simulink model "v01_aa_rii_16r4t11f_ver117sa"  (Simulink 7.6)
settings: Solver=ode45  StartTime=0.0  StopTime=128000  RelTol=1e-3
undefined symbols (not defined in the file or in its folder): sfp
libraries: xbsIndex_r4 x26961, monroe_library x154, xbsReplacements_r4 x78, simulink x32, dspsigops x32, casper_library_misc x30, xps_library x18
(part 18 of 176)

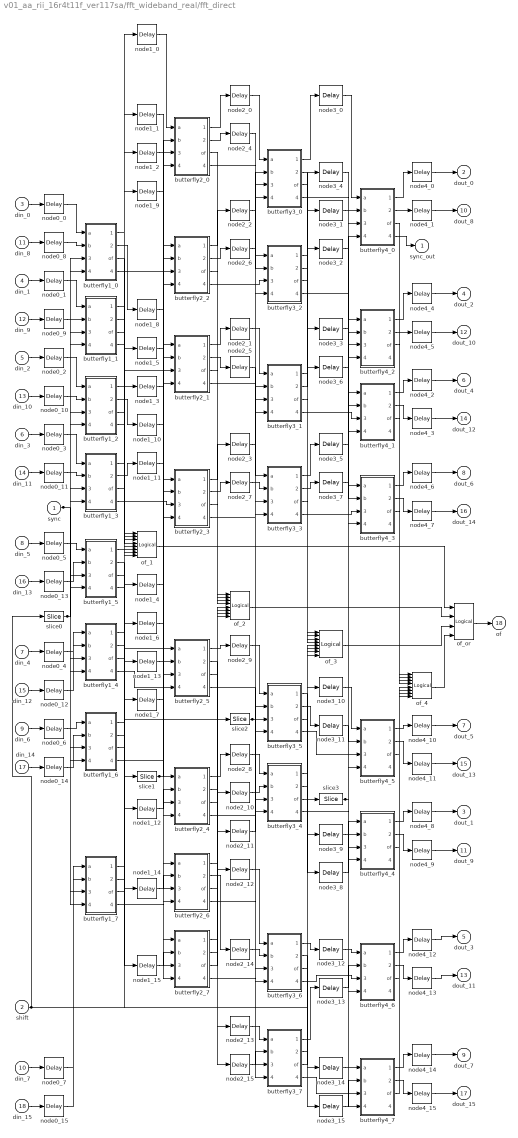
== system v01_aa_rii_16r4t11f_ver117sa/fft_wideband_real/fft_direct ==
  sync : Inport
  shift : Inport  Port=2
  din_0 : Inport  Port=3
  din_1 : Inport  Port=4
  din_2 : Inport  Port=5
  din_3 : Inport  Port=6
  din_4 : Inport  Port=7
  din_5 : Inport  Port=8
  din_6 : Inport  Port=9
  din_7 : Inport  Port=10
  din_8 : Inport  Port=11
  din_9 : Inport  Port=12
  din_10 : Inport  Port=13
  din_11 : Inport  Port=14
  din_12 : Inport  Port=15
  din_13 : Inport  Port=16
  din_14 : Inport  Port=17
  din_15 : Inport  Port=18
  butterfly1_0 : Subsystem
      settings: AttributesFormatString=|
      | twiddle_general_4mult
      | coeffs in BRAM
      | Bit growth:0
  butterfly1_1 : Subsystem
      settings: AttributesFormatString=|
      | twiddle_general_4mult
      | coeffs in BRAM
      | Bit growth:0
  butterfly1_2 : Subsystem
      settings: AttributesFormatString=|
      | twiddle_general_4mult
      | coeffs in BRAM
      | Bit growth:0
  butterfly1_3 : Subsystem
      settings: AttributesFormatString=|
      | twiddle_general_4mult
      | coeffs in BRAM
      | Bit growth:0
  butterfly1_4 : Subsystem
      settings: AttributesFormatString=|
      | twiddle_general_4mult
      | coeffs in BRAM
      | Bit growth:0
  butterfly1_5 : Subsystem
      settings: AttributesFormatString=|
      | twiddle_general_4mult
      | coeffs in BRAM
      | Bit growth:0
  butterfly1_6 : Subsystem
      settings: AttributesFormatString=|
      | twiddle_general_4mult
      | coeffs in BRAM
      | Bit growth:0
  butterfly1_7 : Subsystem
      settings: AttributesFormatString=|
      | twiddle_general_4mult
      | coeffs in BRAM
      | Bit growth:0
  butterfly2_0 : Subsystem
      settings: AttributesFormatString=|
      | twiddle_general_4mult
      | coeffs in BRAM
      | Bit growth:0
  butterfly2_1 : Subsystem
      settings: AttributesFormatString=|
      | twiddle_general_4mult
      | coeffs in BRAM
      | Bit growth:0
  butterfly2_2 : Subsystem
      settings: AttributesFormatString=|
      | twiddle_general_4mult
      | coeffs in BRAM
      | Bit growth:0
  butterfly2_3 : Subsystem
      settings: AttributesFormatString=|
      | twiddle_general_4mult
      | coeffs in BRAM
      | Bit growth:0
  butterfly2_4 : Subsystem
      settings: AttributesFormatString=|
      | twiddle_general_4mult
      | coeffs in BRAM
      | Bit growth:0
  butterfly2_5 : Subsystem
      settings: AttributesFormatString=|
      | twiddle_general_4mult
      | coeffs in BRAM
      | Bit growth:0
  butterfly2_6 : Subsystem
      settings: AttributesFormatString=|
      | twiddle_general_4mult
      | coeffs in BRAM
      | Bit growth:0
  butterfly2_7 : Subsystem
      settings: AttributesFormatString=|
      | twiddle_general_4mult
      | coeffs in BRAM
      | Bit growth:0
  butterfly3_0 : Subsystem
      settings: AttributesFormatString=|
      | twiddle_general_4mult
      | coeffs in BRAM
      | Bit growth:0
  butterfly3_1 : Subsystem
      settings: AttributesFormatString=|
      | twiddle_general_4mult
      | coeffs in BRAM
      | Bit growth:0
  butterfly3_2 : Subsystem
      settings: AttributesFormatString=|
      | twiddle_general_4mult
      | coeffs in BRAM
      | Bit growth:0
  butterfly3_3 : Subsystem
      settings: AttributesFormatString=|
      | twiddle_general_4mult
      | coeffs in BRAM
      | Bit growth:0
  butterfly3_4 : Subsystem
      settings: AttributesFormatString=|
      | twiddle_general_4mult
      | coeffs in BRAM
      | Bit growth:0
  butterfly3_5 : Subsystem
      settings: AttributesFormatString=|
      | twiddle_general_4mult
      | coeffs in BRAM
      | Bit growth:0
  butterfly3_6 : Subsystem
      settings: AttributesFormatString=|
      | twiddle_general_4mult
      | coeffs in BRAM
      | Bit growth:0
  butterfly3_7 : Subsystem
      settings: AttributesFormatString=|
      | twiddle_general_4mult
      | coeffs in BRAM
      | Bit growth:0
  butterfly4_0 : Subsystem
      settings: AttributesFormatString=|
      | twiddle_general_4mult
      | coeffs in BRAM
      | Bit growth:0
  butterfly4_1 : Subsystem
      settings: AttributesFormatString=|
      | twiddle_general_4mult
      | coeffs in BRAM
      | Bit growth:0
  butterfly4_2 : Subsystem
      settings: AttributesFormatString=|
      | twiddle_general_4mult
      | coeffs in BRAM
      | Bit growth:0
  butterfly4_3 : Subsystem
      settings: AttributesFormatString=|
      | twiddle_general_4mult
      | coeffs in BRAM
      | Bit growth:0
  butterfly4_4 : Subsystem
      settings: AttributesFormatString=|
      | twiddle_general_4mult
      | coeffs in BRAM
      | Bit growth:0
  butterfly4_5 : Subsystem
      settings: AttributesFormatString=|
      | twiddle_general_4mult
      | coeffs in BRAM
      | Bit growth:0
  butterfly4_6 : Subsystem
      settings: AttributesFormatString=|
      | twiddle_general_4mult
      | coeffs in BRAM
      | Bit growth:0
  butterfly4_7 : Subsystem
      settings: AttributesFormatString=|
      | twiddle_general_4mult
      | coeffs in BRAM
      | Bit growth:0
  node0_{0..15} (16 alike) : xbsIndex_r4/Delay  rst=off  infoeditControl=Selection of Reset will increase slice count due to use of real FFs and instead of SRLs  en=off  latency=0  dbl_ovrd=off  reg_retiming=off  block_type=delay
  node1_{0..15} (16 alike) : xbsIndex_r4/Delay  rst=off  infoeditControl=Selection of Reset will increase slice count due to use of real FFs and instead of SRLs  en=off  latency=0  dbl_ovrd=off  reg_retiming=off  block_type=delay
  node2_{0..15} (16 alike) : xbsIndex_r4/Delay  rst=off  infoeditControl=Selection of Reset will increase slice count due to use of real FFs and instead of SRLs  en=off  latency=0  dbl_ovrd=off  reg_retiming=off  block_type=delay
  node3_{0..15} (16 alike) : xbsIndex_r4/Delay  rst=off  infoeditControl=Selection of Reset will increase slice count due to use of real FFs and instead of SRLs  en=off  latency=0  dbl_ovrd=off  reg_retiming=off  block_type=delay
  node4_{0..15} (16 alike) : xbsIndex_r4/Delay  rst=off  infoeditControl=Selection of Reset will increase slice count due to use of real FFs and instead of SRLs  en=off  latency=0  dbl_ovrd=off  reg_retiming=off  block_type=delay
  of_{1..4} (4 alike) : xbsIndex_r4/Logical  logical_function=OR  inputs=8  en=off  latency=1  precision=Full  arith_type=Unsigned  n_bits=16  bin_pt=0  align_bp=on  dbl_ovrd=off  block_type=logical
  of_or : xbsIndex_r4/Logical  logical_function=OR  inputs=4  en=off  latency=0  precision=Full  arith_type=Unsigned  n_bits=16  bin_pt=0  align_bp=on  dbl_ovrd=off  block_type=logical
  slice0 : xbsIndex_r4/Slice  nbits=1  boolean_output=on  mode=Lower Bit Location + Width  bit1=0  base1=MSB of Input  bit0=0  base0=LSB of Input  dbl_ovrd=off  block_type=slice
  slice1 : xbsIndex_r4/Slice  nbits=1  boolean_output=on  mode=Lower Bit Location + Width  bit1=0  base1=MSB of Input  bit0=1  base0=LSB of Input  dbl_ovrd=off  block_type=slice
  slice2 : xbsIndex_r4/Slice  nbits=1  boolean_output=on  mode=Lower Bit Location + Width  bit1=0  base1=MSB of Input  bit0=2  base0=LSB of Input  dbl_ovrd=off  block_type=slice
  slice3 : xbsIndex_r4/Slice  nbits=1  boolean_output=on  mode=Lower Bit Location + Width  bit1=0  base1=MSB of Input  bit0=3  base0=LSB of Input  dbl_ovrd=off  block_type=slice
  sync_out : Outport
  dout_0 : Outport  Port=2
  dout_1 : Outport  Port=3
  dout_2 : Outport  Port=4
  dout_3 : Outport  Port=5
  dout_4 : Outport  Port=6
  dout_5 : Outport  Port=7
  dout_6 : Outport  Port=8
  dout_7 : Outport  Port=9
  dout_8 : Outport  Port=10
  dout_9 : Outport  Port=11
  dout_10 : Outport  Port=12
  dout_11 : Outport  Port=13
  dout_12 : Outport  Port=14
  dout_13 : Outport  Port=15
  dout_14 : Outport  Port=16
  dout_15 : Outport  Port=17
  of : Outport  Port=18
  wires:
  shift/1 -> slice3/1, slice2/1, slice1/1, slice0/1
  sync/1 -> butterfly1_7/3(sync), butterfly1_6/3(sync), butterfly1_5/3(sync), butterfly1_4/3(sync), butterfly1_3/3(sync), butterfly1_2/3(sync), butterfly1_1/3(sync), butterfly1_0/3(sync)
  slice0/1 -> butterfly1_7/4(shift), butterfly1_6/4(shift), butterfly1_5/4(shift), butterfly1_4/4(shift), butterfly1_3/4(shift), butterfly1_2/4(shift), butterfly1_1/4(shift), butterfly1_0/4(shift)
  slice1/1 -> butterfly2_7/4(shift), butterfly2_6/4(shift), butterfly2_5/4(shift), butterfly2_4/4(shift), butterfly2_3/4(shift), butterfly2_2/4(shift), butterfly2_1/4(shift), butterfly2_0/4(shift)
  slice2/1 -> butterfly3_7/4(shift), butterfly3_6/4(shift), butterfly3_5/4(shift), butterfly3_4/4(shift), butterfly3_3/4(shift), butterfly3_2/4(shift), butterfly3_1/4(shift), butterfly3_0/4(shift)
  slice3/1 -> butterfly4_7/4(shift), butterfly4_6/4(shift), butterfly4_5/4(shift), butterfly4_4/4(shift), butterfly4_3/4(shift), butterfly4_2/4(shift), butterfly4_1/4(shift), butterfly4_0/4(shift)
  butterfly4_0/4(sync_out) -> sync_out/1
  of_or/1 -> of/1
  of_4/1 -> of_or/4
  of_3/1 -> of_or/3
  of_2/1 -> of_or/2
  of_1/1 -> of_or/1
  butterfly4_7/3(of) -> of_4/8
  butterfly4_6/3(of) -> of_4/7
  butterfly4_5/3(of) -> of_4/6
  butterfly4_4/3(of) -> of_4/5
  butterfly4_3/3(of) -> of_4/4
  butterfly4_2/3(of) -> of_4/3
  butterfly4_1/3(of) -> of_4/2
  butterfly4_0/3(of) -> of_4/1
  butterfly4_7/2(a-bw) -> node4_15/1
  butterfly4_7/1(a+bw) -> node4_14/1
  butterfly3_7/4(sync_out) -> butterfly4_7/3(sync)
  node3_15/1 -> butterfly4_7/2(b)
  node3_14/1 -> butterfly4_7/1(a)
  butterfly4_6/2(a-bw) -> node4_13/1
  butterfly4_6/1(a+bw) -> node4_12/1
  butterfly3_6/4(sync_out) -> butterfly4_6/3(sync)
  node3_13/1 -> butterfly4_6/2(b)
  node3_12/1 -> butterfly4_6/1(a)
  butterfly4_5/2(a-bw) -> node4_11/1
  butterfly4_5/1(a+bw) -> node4_10/1
  butterfly3_5/4(sync_out) -> butterfly4_5/3(sync)
  node3_11/1 -> butterfly4_5/2(b)
  node3_10/1 -> butterfly4_5/1(a)
  butterfly4_4/2(a-bw) -> node4_9/1
  butterfly4_4/1(a+bw) -> node4_8/1
  butterfly3_4/4(sync_out) -> butterfly4_4/3(sync)
  node3_9/1 -> butterfly4_4/2(b)
  node3_8/1 -> butterfly4_4/1(a)
  butterfly4_3/2(a-bw) -> node4_7/1
  butterfly4_3/1(a+bw) -> node4_6/1
  butterfly3_3/4(sync_out) -> butterfly4_3/3(sync)
  node3_7/1 -> butterfly4_3/2(b)
  node3_6/1 -> butterfly4_3/1(a)
  butterfly4_2/2(a-bw) -> node4_5/1
  butterfly4_2/1(a+bw) -> node4_4/1
  butterfly3_2/4(sync_out) -> butterfly4_2/3(sync)
  node3_5/1 -> butterfly4_2/2(b)
  node3_4/1 -> butterfly4_2/1(a)
  butterfly4_1/2(a-bw) -> node4_3/1
  butterfly4_1/1(a+bw) -> node4_2/1
  butterfly3_1/4(sync_out) -> butterfly4_1/3(sync)
  node3_3/1 -> butterfly4_1/2(b)
  node3_2/1 -> butterfly4_1/1(a)
  butterfly4_0/2(a-bw) -> node4_1/1
  butterfly4_0/1(a+bw) -> node4_0/1
  butterfly3_0/4(sync_out) -> butterfly4_0/3(sync)
  node3_1/1 -> butterfly4_0/2(b)
  node3_0/1 -> butterfly4_0/1(a)
  butterfly3_7/3(of) -> of_3/8
  butterfly3_6/3(of) -> of_3/7
  butterfly3_5/3(of) -> of_3/6
  butterfly3_4/3(of) -> of_3/5
  butterfly3_3/3(of) -> of_3/4
  butterfly3_2/3(of) -> of_3/3
  butterfly3_1/3(of) -> of_3/2
  butterfly3_0/3(of) -> of_3/1
  butterfly3_7/2(a-bw) -> node3_15/1
  butterfly3_7/1(a+bw) -> node3_13/1
  butterfly2_7/4(sync_out) -> butterfly3_7/3(sync)
  node2_15/1 -> butterfly3_7/2(b)
  node2_13/1 -> butterfly3_7/1(a)
  butterfly3_6/2(a-bw) -> node3_14/1
  butterfly3_6/1(a+bw) -> node3_12/1
  butterfly2_6/4(sync_out) -> butterfly3_6/3(sync)
  node2_14/1 -> butterfly3_6/2(b)
  node2_12/1 -> butterfly3_6/1(a)
  butterfly3_5/2(a-bw) -> node3_11/1
  butterfly3_5/1(a+bw) -> node3_9/1
  butterfly2_5/4(sync_out) -> butterfly3_5/3(sync)
  node2_11/1 -> butterfly3_5/2(b)
  node2_9/1 -> butterfly3_5/1(a)
  butterfly3_4/2(a-bw) -> node3_10/1
  butterfly3_4/1(a+bw) -> node3_8/1
  butterfly2_4/4(sync_out) -> butterfly3_4/3(sync)
  node2_10/1 -> butterfly3_4/2(b)
  node2_8/1 -> butterfly3_4/1(a)
  butterfly3_3/2(a-bw) -> node3_7/1
  butterfly3_3/1(a+bw) -> node3_5/1
  butterfly2_3/4(sync_out) -> butterfly3_3/3(sync)
  node2_7/1 -> butterfly3_3/2(b)
  node2_5/1 -> butterfly3_3/1(a)
  butterfly3_2/2(a-bw) -> node3_6/1
  butterfly3_2/1(a+bw) -> node3_4/1
  butterfly2_2/4(sync_out) -> butterfly3_2/3(sync)
  node2_6/1 -> butterfly3_2/2(b)
  node2_4/1 -> butterfly3_2/1(a)
  butterfly3_1/2(a-bw) -> node3_3/1
  butterfly3_1/1(a+bw) -> node3_1/1
  butterfly2_1/4(sync_out) -> butterfly3_1/3(sync)
  node2_3/1 -> butterfly3_1/2(b)
  node2_1/1 -> butterfly3_1/1(a)
  butterfly3_0/2(a-bw) -> node3_2/1
  butterfly3_0/1(a+bw) -> node3_0/1
  butterfly2_0/4(sync_out) -> butterfly3_0/3(sync)
  node2_2/1 -> butterfly3_0/2(b)
  node2_0/1 -> butterfly3_0/1(a)
  butterfly2_7/3(of) -> of_2/8
  butterfly2_6/3(of) -> of_2/7
  butterfly2_5/3(of) -> of_2/6
  butterfly2_4/3(of) -> of_2/5
  butterfly2_3/3(of) -> of_2/4
  butterfly2_2/3(of) -> of_2/3
  butterfly2_1/3(of) -> of_2/2
  butterfly2_0/3(of) -> of_2/1
  butterfly2_7/2(a-bw) -> node2_15/1
  butterfly2_7/1(a+bw) -> node2_11/1
  butterfly1_7/4(sync_out) -> butterfly2_7/3(sync)
  node1_15/1 -> butterfly2_7/2(b)
  node1_11/1 -> butterfly2_7/1(a)
  butterfly2_6/2(a-bw) -> node2_14/1
  butterfly2_6/1(a+bw) -> node2_10/1
  butterfly1_6/4(sync_out) -> butterfly2_6/3(sync)
  node1_14/1 -> butterfly2_6/2(b)
  node1_10/1 -> butterfly2_6/1(a)
  butterfly2_5/2(a-bw) -> node2_13/1
  butterfly2_5/1(a+bw) -> node2_9/1
  butterfly1_5/4(sync_out) -> butterfly2_5/3(sync)
  node1_13/1 -> butterfly2_5/2(b)
  node1_9/1 -> butterfly2_5/1(a)
  butterfly2_4/2(a-bw) -> node2_12/1
  butterfly2_4/1(a+bw) -> node2_8/1
  butterfly1_4/4(sync_out) -> butterfly2_4/3(sync)
  node1_12/1 -> butterfly2_4/2(b)
  node1_8/1 -> butterfly2_4/1(a)
  butterfly2_3/2(a-bw) -> node2_7/1
  butterfly2_3/1(a+bw) -> node2_3/1
  butterfly1_3/4(sync_out) -> butterfly2_3/3(sync)
  node1_7/1 -> butterfly2_3/2(b)
  node1_3/1 -> butterfly2_3/1(a)
  butterfly2_2/2(a-bw) -> node2_6/1
  butterfly2_2/1(a+bw) -> node2_2/1
  butterfly1_2/4(sync_out) -> butterfly2_2/3(sync)
  node1_6/1 -> butterfly2_2/2(b)
  node1_2/1 -> butterfly2_2/1(a)
  butterfly2_1/2(a-bw) -> node2_5/1
  butterfly2_1/1(a+bw) -> node2_1/1
  butterfly1_1/4(sync_out) -> butterfly2_1/3(sync)
  node1_5/1 -> butterfly2_1/2(b)
  node1_1/1 -> butterfly2_1/1(a)
  butterfly2_0/2(a-bw) -> node2_4/1
  butterfly2_0/1(a+bw) -> node2_0/1
  butterfly1_0/4(sync_out) -> butterfly2_0/3(sync)
  node1_4/1 -> butterfly2_0/2(b)
  node1_0/1 -> butterfly2_0/1(a)
  butterfly1_7/3(of) -> of_1/8
  butterfly1_6/3(of) -> of_1/7
  butterfly1_5/3(of) -> of_1/6
  butterfly1_4/3(of) -> of_1/5
  butterfly1_3/3(of) -> of_1/4
  butterfly1_2/3(of) -> of_1/3
  butterfly1_1/3(of) -> of_1/2
  butterfly1_0/3(of) -> of_1/1
  butterfly1_7/2(a-bw) -> node1_15/1
  butterfly1_7/1(a+bw) -> node1_7/1
  node0_15/1 -> butterfly1_7/2(b)
  node0_7/1 -> butterfly1_7/1(a)
  butterfly1_6/2(a-bw) -> node1_14/1
  butterfly1_6/1(a+bw) -> node1_6/1
  node0_14/1 -> butterfly1_6/2(b)
  node0_6/1 -> butterfly1_6/1(a)
  butterfly1_5/2(a-bw) -> node1_13/1
  butterfly1_5/1(a+bw) -> node1_5/1
  node0_13/1 -> butterfly1_5/2(b)
  node0_5/1 -> butterfly1_5/1(a)
  butterfly1_4/2(a-bw) -> node1_12/1
  butterfly1_4/1(a+bw) -> node1_4/1
  node0_12/1 -> butterfly1_4/2(b)
  node0_4/1 -> butterfly1_4/1(a)
  butterfly1_3/2(a-bw) -> node1_11/1
  butterfly1_3/1(a+bw) -> node1_3/1
  node0_11/1 -> butterfly1_3/2(b)
  node0_3/1 -> butterfly1_3/1(a)
  butterfly1_2/2(a-bw) -> node1_10/1
  butterfly1_2/1(a+bw) -> node1_2/1
  node0_10/1 -> butterfly1_2/2(b)
  node0_2/1 -> butterfly1_2/1(a)
  butterfly1_1/2(a-bw) -> node1_9/1
  butterfly1_1/1(a+bw) -> node1_1/1
  node0_9/1 -> butterfly1_1/2(b)
  node0_1/1 -> butterfly1_1/1(a)
  butterfly1_0/2(a-bw) -> node1_8/1
  butterfly1_0/1(a+bw) -> node1_0/1
  node0_8/1 -> butterfly1_0/2(b)
  node0_0/1 -> butterfly1_0/1(a)
  node4_15/1 -> dout_15/1
  node4_14/1 -> dout_7/1
  node4_13/1 -> dout_11/1
  node4_12/1 -> dout_3/1
  node4_11/1 -> dout_13/1
  node4_10/1 -> dout_5/1
  node4_9/1 -> dout_9/1
  node4_8/1 -> dout_1/1
  node4_7/1 -> dout_14/1
  node4_6/1 -> dout_6/1
  node4_5/1 -> dout_10/1
  node4_4/1 -> dout_2/1
  node4_3/1 -> dout_12/1
  node4_2/1 -> dout_4/1
  node4_1/1 -> dout_8/1
  node4_0/1 -> dout_0/1
  din_15/1 -> node0_15/1
  din_14/1 -> node0_14/1
  din_13/1 -> node0_13/1
  din_12/1 -> node0_12/1
  din_11/1 -> node0_11/1
  din_10/1 -> node0_10/1
  din_9/1 -> node0_9/1
  din_8/1 -> node0_8/1
  din_7/1 -> node0_7/1
  din_6/1 -> node0_6/1
  din_5/1 -> node0_5/1
  din_4/1 -> node0_4/1
  din_3/1 -> node0_3/1
  din_2/1 -> node0_2/1
  din_1/1 -> node0_1/1
  din_0/1 -> node0_0/1

== system v01_aa_rii_16r4t11f_ver117sa/fft_wideband_real/fft_unscrambler ==
  sync : Inport
  In1 : Inport  Port=2
  In2 : Inport  Port=3
  In3 : Inport  Port=4
  In4 : Inport  Port=5
  In5 : Inport  Port=6
  In6 : Inport  Port=7
  In7 : Inport  Port=8
  In8 : Inport  Port=9
  const : xbsIndex_r4/Constant  const=1  gui_display_data_type=Fixed-point  arith_type=Boolean  n_bits=1  bin_pt=0  preci_type=Single  exp_width=8  frac_width=24  explicit_period=on  period=1  equ=P=C  opselect=C  inp2=PCIN>>17  opr=+  inp1=P  carry=CIN  dbl_ovrd=off  block_type=constant
  reorder1 : Subsystem
      settings: AttributesFormatString=order=7
  square_transposer1 : Subsystem
      settings: AttributesFormatString=n_inputs=3
  sync_out : Outport
  Out1 : Outport  Port=2
  Out2 : Outport  Port=3
  Out3 : Outport  Port=4
  Out4 : Outport  Port=5
  Out5 : Outport  Port=6
  Out6 : Outport  Port=7
  Out7 : Outport  Port=8
  Out8 : Outport  Port=9
  wires:
  square_transposer1/9(Out8) -> reorder1/10(din7)  "xlsub2_reorder_In8"
  square_transposer1/8(Out7) -> reorder1/9(din6)  "xlsub2_reorder_In7"
  square_transposer1/7(Out6) -> reorder1/8(din5)  "xlsub2_reorder_In6"
  square_transposer1/6(Out5) -> reorder1/7(din4)  "xlsub2_reorder_In5"
  square_transposer1/5(Out4) -> reorder1/6(din3)  "xlsub2_reorder_In4"
  square_transposer1/4(Out3) -> reorder1/5(din2)  "xlsub2_reorder_In3"
  square_transposer1/3(Out2) -> reorder1/4(din1)  "xlsub2_reorder_In2"
  square_transposer1/2(Out1) -> reorder1/3(din0)  "xlsub2_reorder_In1"
  square_transposer1/1(sync_out) -> reorder1/1(sync)
  In8/1 -> square_transposer1/9(In8)
  In7/1 -> square_transposer1/8(In7)
  In6/1 -> square_transposer1/7(In6)
  In5/1 -> square_transposer1/6(In5)
  In4/1 -> square_transposer1/5(In4)
  In3/1 -> square_transposer1/4(In3)
  In2/1 -> square_transposer1/3(In2)
  In1/1 -> square_transposer1/2(In1)
  sync/1 -> square_transposer1/1(sync)
  reorder1/10(dout7) -> Out8/1
  reorder1/9(dout6) -> Out7/1
  reorder1/8(dout5) -> Out6/1
  reorder1/7(dout4) -> Out5/1
  reorder1/6(dout3) -> Out4/1
  reorder1/5(dout2) -> Out3/1
  reorder1/4(dout1) -> Out2/1
  reorder1/3(dout0) -> Out1/1
  reorder1/1(sync_out) -> sync_out/1
  const/1 -> reorder1/2(en)

== system v01_aa_rii_16r4t11f_ver117sa/fft_wideband_real/in_del_4x0pol1 ==
  d : Inport
  q : Outport
  wires:
  d/1 -> q/1

== system v01_aa_rii_16r4t11f_ver117sa/fft_wideband_real/in_del_4x0pol2 ==
  d : Inport
  q : Outport
  wires:
  d/1 -> q/1

== system v01_aa_rii_16r4t11f_ver117sa/fft_wideband_real/in_del_4x0pol3 ==
  d : Inport
  q : Outport
  wires:
  d/1 -> q/1

== system v01_aa_rii_16r4t11f_ver117sa/fft_wideband_real/in_del_4x0pol4 ==
  d : Inport
  q : Outport
  wires:
  d/1 -> q/1

== system v01_aa_rii_16r4t11f_ver117sa/fft_wideband_real/in_del_4x1pol1 ==
  d : Inport
  q : Outport
  wires:
  d/1 -> q/1

== system v01_aa_rii_16r4t11f_ver117sa/fft_wideband_real/in_del_4x1pol2 ==
  d : Inport
  q : Outport
  wires:
  d/1 -> q/1

== system v01_aa_rii_16r4t11f_ver117sa/fft_wideband_real/in_del_4x1pol3 ==
  d : Inport
  q : Outport
  wires:
  d/1 -> q/1

== system v01_aa_rii_16r4t11f_ver117sa/fft_wideband_real/in_del_4x1pol4 ==
  d : Inport
  q : Outport
  wires:
  d/1 -> q/1

== system v01_aa_rii_16r4t11f_ver117sa/fft_wideband_real/in_del_4x2pol1 ==
  d : Inport
  q : Outport
  wires:
  d/1 -> q/1

== system v01_aa_rii_16r4t11f_ver117sa/fft_wideband_real/in_del_4x2pol2 ==
  d : Inport
  q : Outport
  wires:
  d/1 -> q/1

== system v01_aa_rii_16r4t11f_ver117sa/fft_wideband_real/in_del_4x2pol3 ==
  d : Inport
  q : Outport
  wires:
  d/1 -> q/1

== system v01_aa_rii_16r4t11f_ver117sa/fft_wideband_real/in_del_4x2pol4 ==
  d : Inport
  q : Outport
  wires:
  d/1 -> q/1

== system v01_aa_rii_16r4t11f_ver117sa/fft_wideband_real/in_del_4x3pol1 ==
  d : Inport
  q : Outport
  wires:
  d/1 -> q/1

== system v01_aa_rii_16r4t11f_ver117sa/fft_wideband_real/in_del_4x3pol2 ==
  d : Inport
  q : Outport
  wires:
  d/1 -> q/1

== system v01_aa_rii_16r4t11f_ver117sa/fft_wideband_real/in_del_4x3pol3 ==
  d : Inport
  q : Outport
  wires:
  d/1 -> q/1

== system v01_aa_rii_16r4t11f_ver117sa/fft_wideband_real/in_del_4x3pol4 ==
  d : Inport
  q : Outport
  wires:
  d/1 -> q/1

== system v01_aa_rii_16r4t11f_ver117sa/fft_wideband_real/in_del_sync_4x0 ==
  d : Inport
  q : Outport
  wires:
  d/1 -> q/1

== system v01_aa_rii_16r4t11f_ver117sa/fft_wideband_real/in_del_sync_4x1 ==
  d : Inport
  q : Outport
  wires:
  d/1 -> q/1

== system v01_aa_rii_16r4t11f_ver117sa/fft_wideband_real/in_del_sync_4x2 ==
  d : Inport
  q : Outport
  wires:
  d/1 -> q/1

== system v01_aa_rii_16r4t11f_ver117sa/fft_wideband_real/in_del_sync_4x3 ==
  d : Inport
  q : Outport
  wires:
  d/1 -> q/1

== system v01_aa_rii_16r4t11f_ver117sa/fft_wideband_real/out_del_4x0pol1 ==
  d : Inport
  q : Outport
  wires:
  d/1 -> q/1

== system v01_aa_rii_16r4t11f_ver117sa/fft_wideband_real/out_del_4x0pol2 ==
  d : Inport
  q : Outport
  wires:
  d/1 -> q/1

== system v01_aa_rii_16r4t11f_ver117sa/fft_wideband_real/out_del_4x0pol3 ==
  d : Inport
  q : Outport
  wires:
  d/1 -> q/1

== system v01_aa_rii_16r4t11f_ver117sa/fft_wideband_real/out_del_4x0pol4 ==
  d : Inport
  q : Outport
  wires:
  d/1 -> q/1

== system v01_aa_rii_16r4t11f_ver117sa/fft_wideband_real/out_del_4x1pol1 ==
  d : Inport
  q : Outport
  wires:
  d/1 -> q/1

== system v01_aa_rii_16r4t11f_ver117sa/fft_wideband_real/out_del_4x1pol2 ==
  d : Inport
  q : Outport
  wires:
  d/1 -> q/1

== system v01_aa_rii_16r4t11f_ver117sa/fft_wideband_real/out_del_4x1pol3 ==
  d : Inport
  q : Outport
  wires:
  d/1 -> q/1

== system v01_aa_rii_16r4t11f_ver117sa/fft_wideband_real/out_del_4x1pol4 ==
  d : Inport
  q : Outport
  wires:
  d/1 -> q/1

== system v01_aa_rii_16r4t11f_ver117sa/fft_wideband_real/out_del_4x2pol1 ==
  d : Inport
  q : Outport
  wires:
  d/1 -> q/1

== system v01_aa_rii_16r4t11f_ver117sa/fft_wideband_real/out_del_4x2pol2 ==
  d : Inport
  q : Outport
  wires:
  d/1 -> q/1

== system v01_aa_rii_16r4t11f_ver117sa/fft_wideband_real/out_del_4x2pol3 ==
  d : Inport
  q : Outport
  wires:
  d/1 -> q/1

== system v01_aa_rii_16r4t11f_ver117sa/fft_wideband_real/out_del_4x2pol4 ==
  d : Inport
  q : Outport
  wires:
  d/1 -> q/1

== system v01_aa_rii_16r4t11f_ver117sa/fft_wideband_real/out_del_4x3pol1 ==
  d : Inport
  q : Outport
  wires:
  d/1 -> q/1

== system v01_aa_rii_16r4t11f_ver117sa/fft_wideband_real/out_del_4x3pol2 ==
  d : Inport
  q : Outport
  wires:
  d/1 -> q/1

== system v01_aa_rii_16r4t11f_ver117sa/fft_wideband_real/out_del_4x3pol3 ==
  d : Inport
  q : Outport
  wires:
  d/1 -> q/1

== system v01_aa_rii_16r4t11f_ver117sa/fft_wideband_real/out_del_4x3pol4 ==
  d : Inport
  q : Outport
  wires:
  d/1 -> q/1

== system v01_aa_rii_16r4t11f_ver117sa/fft_wideband_real/out_del_sync_4x0 ==
  d : Inport
  q : Outport
  wires:
  d/1 -> q/1

== system v01_aa_rii_16r4t11f_ver117sa/fft_wideband_real1/fft_biplex_real_4x0 ==
  sync : Inport
  shift : Inport  Port=2
  pol1 : Inport  Port=3
  pol2 : Inport  Port=4
  pol3 : Inport  Port=5
  pol4 : Inport  Port=6
  bi_real_unscr_4x : Subsystem
  biplex_core : Subsystem
      settings: AttributesFormatString=|
      | 7 stages
      | reduce logic
      | Virtex5
  ri_to_c_pol1 : Subsystem
  ri_to_c_pol2 : Subsystem
  sync_out : Outport
  pol1_out : Outport  Port=2
  pol2_out : Outport  Port=3
  pol3_out : Outport  Port=4
  pol4_out : Outport  Port=5
  of : Outport  Port=6
  wires:
  bi_real_unscr_4x/5(pol4_out) -> pol4_out/1
  bi_real_unscr_4x/4(pol3_out) -> pol3_out/1
  bi_real_unscr_4x/3(pol2_out) -> pol2_out/1
  bi_real_unscr_4x/2(pol1_out) -> pol1_out/1
  bi_real_unscr_4x/1(sync_out) -> sync_out/1
  biplex_core/3(out2) -> bi_real_unscr_4x/3(odd)
  biplex_core/2(out1) -> bi_real_unscr_4x/2(even)
  biplex_core/1(sync_out) -> bi_real_unscr_4x/1(sync)
  biplex_core/4(of) -> of/1
  ri_to_c_pol2/1(c) -> biplex_core/4(pol2)
  ri_to_c_pol1/1(c) -> biplex_core/3(pol1)
  shift/1 -> biplex_core/2(shift)
  sync/1 -> biplex_core/1(sync)
  pol4/1 -> ri_to_c_pol2/2(im)
  pol3/1 -> ri_to_c_pol2/1(re)
  pol2/1 -> ri_to_c_pol1/2(im)
  pol1/1 -> ri_to_c_pol1/1(re)

== system v01_aa_rii_16r4t11f_ver117sa/fft_wideband_real1/fft_biplex_real_4x1 ==
  sync : Inport
  shift : Inport  Port=2
  pol1 : Inport  Port=3
  pol2 : Inport  Port=4
  pol3 : Inport  Port=5
  pol4 : Inport  Port=6
  bi_real_unscr_4x : Subsystem
  biplex_core : Subsystem
      settings: AttributesFormatString=|
      | 7 stages
      | reduce logic
      | Virtex5
  ri_to_c_pol1 : Subsystem
  ri_to_c_pol2 : Subsystem
  sync_out : Outport
  pol1_out : Outport  Port=2
  pol2_out : Outport  Port=3
  pol3_out : Outport  Port=4
  pol4_out : Outport  Port=5
  of : Outport  Port=6
  wires:
  bi_real_unscr_4x/5(pol4_out) -> pol4_out/1
  bi_real_unscr_4x/4(pol3_out) -> pol3_out/1
  bi_real_unscr_4x/3(pol2_out) -> pol2_out/1
  bi_real_unscr_4x/2(pol1_out) -> pol1_out/1
  bi_real_unscr_4x/1(sync_out) -> sync_out/1
  biplex_core/3(out2) -> bi_real_unscr_4x/3(odd)
  biplex_core/2(out1) -> bi_real_unscr_4x/2(even)
  biplex_core/1(sync_out) -> bi_real_unscr_4x/1(sync)
  biplex_core/4(of) -> of/1
  ri_to_c_pol2/1(c) -> biplex_core/4(pol2)
  ri_to_c_pol1/1(c) -> biplex_core/3(pol1)
  shift/1 -> biplex_core/2(shift)
  sync/1 -> biplex_core/1(sync)
  pol4/1 -> ri_to_c_pol2/2(im)
  pol3/1 -> ri_to_c_pol2/1(re)
  pol2/1 -> ri_to_c_pol1/2(im)
  pol1/1 -> ri_to_c_pol1/1(re)

== system v01_aa_rii_16r4t11f_ver117sa/fft_wideband_real1/fft_biplex_real_4x2 ==
  sync : Inport
  shift : Inport  Port=2
  pol1 : Inport  Port=3
  pol2 : Inport  Port=4
  pol3 : Inport  Port=5
  pol4 : Inport  Port=6
  bi_real_unscr_4x : Subsystem
  biplex_core : Subsystem
      settings: AttributesFormatString=|
      | 7 stages
      | reduce logic
      | Virtex5
  ri_to_c_pol1 : Subsystem
  ri_to_c_pol2 : Subsystem
  sync_out : Outport
  pol1_out : Outport  Port=2
  pol2_out : Outport  Port=3
  pol3_out : Outport  Port=4
  pol4_out : Outport  Port=5
  of : Outport  Port=6
  wires:
  bi_real_unscr_4x/5(pol4_out) -> pol4_out/1
  bi_real_unscr_4x/4(pol3_out) -> pol3_out/1
  bi_real_unscr_4x/3(pol2_out) -> pol2_out/1
  bi_real_unscr_4x/2(pol1_out) -> pol1_out/1
  bi_real_unscr_4x/1(sync_out) -> sync_out/1
  biplex_core/3(out2) -> bi_real_unscr_4x/3(odd)
  biplex_core/2(out1) -> bi_real_unscr_4x/2(even)
  biplex_core/1(sync_out) -> bi_real_unscr_4x/1(sync)
  biplex_core/4(of) -> of/1
  ri_to_c_pol2/1(c) -> biplex_core/4(pol2)
  ri_to_c_pol1/1(c) -> biplex_core/3(pol1)
  shift/1 -> biplex_core/2(shift)
  sync/1 -> biplex_core/1(sync)
  pol4/1 -> ri_to_c_pol2/2(im)
  pol3/1 -> ri_to_c_pol2/1(re)
  pol2/1 -> ri_to_c_pol1/2(im)
  pol1/1 -> ri_to_c_pol1/1(re)

== system v01_aa_rii_16r4t11f_ver117sa/fft_wideband_real1/fft_biplex_real_4x3 ==
  sync : Inport
  shift : Inport  Port=2
  pol1 : Inport  Port=3
  pol2 : Inport  Port=4
  pol3 : Inport  Port=5
  pol4 : Inport  Port=6
  bi_real_unscr_4x : Subsystem
  biplex_core : Subsystem
      settings: AttributesFormatString=|
      | 7 stages
      | reduce logic
      | Virtex5
  ri_to_c_pol1 : Subsystem
  ri_to_c_pol2 : Subsystem
  sync_out : Outport
  pol1_out : Outport  Port=2
  pol2_out : Outport  Port=3
  pol3_out : Outport  Port=4
  pol4_out : Outport  Port=5
  of : Outport  Port=6
  wires:
  bi_real_unscr_4x/5(pol4_out) -> pol4_out/1
  bi_real_unscr_4x/4(pol3_out) -> pol3_out/1
  bi_real_unscr_4x/3(pol2_out) -> pol2_out/1
  bi_real_unscr_4x/2(pol1_out) -> pol1_out/1
  bi_real_unscr_4x/1(sync_out) -> sync_out/1
  biplex_core/3(out2) -> bi_real_unscr_4x/3(odd)
  biplex_core/2(out1) -> bi_real_unscr_4x/2(even)
  biplex_core/1(sync_out) -> bi_real_unscr_4x/1(sync)
  biplex_core/4(of) -> of/1
  ri_to_c_pol2/1(c) -> biplex_core/4(pol2)
  ri_to_c_pol1/1(c) -> biplex_core/3(pol1)
  shift/1 -> biplex_core/2(shift)
  sync/1 -> biplex_core/1(sync)
  pol4/1 -> ri_to_c_pol2/2(im)
  pol3/1 -> ri_to_c_pol2/1(re)
  pol2/1 -> ri_to_c_pol1/2(im)
  pol1/1 -> ri_to_c_pol1/1(re)

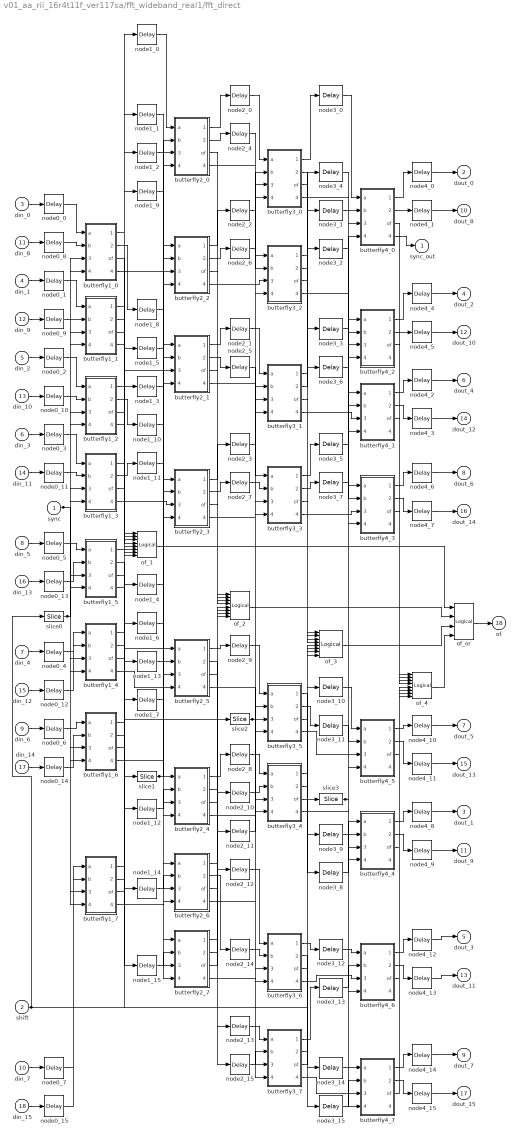
== system v01_aa_rii_16r4t11f_ver117sa/fft_wideband_real1/fft_direct ==
  sync : Inport
  shift : Inport  Port=2
  din_0 : Inport  Port=3
  din_1 : Inport  Port=4
  din_2 : Inport  Port=5
  din_3 : Inport  Port=6
  din_4 : Inport  Port=7
  din_5 : Inport  Port=8
  din_6 : Inport  Port=9
  din_7 : Inport  Port=10
  din_8 : Inport  Port=11
  din_9 : Inport  Port=12
  din_10 : Inport  Port=13
  din_11 : Inport  Port=14
  din_12 : Inport  Port=15
  din_13 : Inport  Port=16
  din_14 : Inport  Port=17
  din_15 : Inport  Port=18
  butterfly1_0 : Subsystem
      settings: AttributesFormatString=|
      | twiddle_general_4mult
      | coeffs in BRAM
      | Bit growth:0
  butterfly1_1 : Subsystem
      settings: AttributesFormatString=|
      | twiddle_general_4mult
      | coeffs in BRAM
      | Bit growth:0
  butterfly1_2 : Subsystem
      settings: AttributesFormatString=|
      | twiddle_general_4mult
      | coeffs in BRAM
      | Bit growth:0
  butterfly1_3 : Subsystem
      settings: AttributesFormatString=|
      | twiddle_general_4mult
      | coeffs in BRAM
      | Bit growth:0
  butterfly1_4 : Subsystem
      settings: AttributesFormatString=|
      | twiddle_general_4mult
      | coeffs in BRAM
      | Bit growth:0
  butterfly1_5 : Subsystem
      settings: AttributesFormatString=|
      | twiddle_general_4mult
      | coeffs in BRAM
      | Bit growth:0
  butterfly1_6 : Subsystem
      settings: AttributesFormatString=|
      | twiddle_general_4mult
      | coeffs in BRAM
      | Bit growth:0
  butterfly1_7 : Subsystem
      settings: AttributesFormatString=|
      | twiddle_general_4mult
      | coeffs in BRAM
      | Bit growth:0
  butterfly2_0 : Subsystem
      settings: AttributesFormatString=|
      | twiddle_general_4mult
      | coeffs in BRAM
      | Bit growth:0
  butterfly2_1 : Subsystem
      settings: AttributesFormatString=|
      | twiddle_general_4mult
      | coeffs in BRAM
      | Bit growth:0
  butterfly2_2 : Subsystem
      settings: AttributesFormatString=|
      | twiddle_general_4mult
      | coeffs in BRAM
      | Bit growth:0
  butterfly2_3 : Subsystem
      settings: AttributesFormatString=|
      | twiddle_general_4mult
      | coeffs in BRAM
      | Bit growth:0
  butterfly2_4 : Subsystem
      settings: AttributesFormatString=|
      | twiddle_general_4mult
      | coeffs in BRAM
      | Bit growth:0
  butterfly2_5 : Subsystem
      settings: AttributesFormatString=|
      | twiddle_general_4mult
      | coeffs in BRAM
      | Bit growth:0
  butterfly2_6 : Subsystem
      settings: AttributesFormatString=|
      | twiddle_general_4mult
      | coeffs in BRAM
      | Bit growth:0
  butterfly2_7 : Subsystem
      settings: AttributesFormatString=|
      | twiddle_general_4mult
      | coeffs in BRAM
      | Bit growth:0
  butterfly3_0 : Subsystem
      settings: AttributesFormatString=|
      | twiddle_general_4mult
      | coeffs in BRAM
      | Bit growth:0
  butterfly3_1 : Subsystem
      settings: AttributesFormatString=|
      | twiddle_general_4mult
      | coeffs in BRAM
      | Bit growth:0
  butterfly3_2 : Subsystem
      settings: AttributesFormatString=|
      | twiddle_general_4mult
      | coeffs in BRAM
      | Bit growth:0
  butterfly3_3 : Subsystem
      settings: AttributesFormatString=|
      | twiddle_general_4mult
      | coeffs in BRAM
      | Bit growth:0
  butterfly3_4 : Subsystem
      settings: AttributesFormatString=|
      | twiddle_general_4mult
      | coeffs in BRAM
      | Bit growth:0
  butterfly3_5 : Subsystem
      settings: AttributesFormatString=|
      | twiddle_general_4mult
      | coeffs in BRAM
      | Bit growth:0
  butterfly3_6 : Subsystem
      settings: AttributesFormatString=|
      | twiddle_general_4mult
      | coeffs in BRAM
      | Bit growth:0
  butterfly3_7 : Subsystem
      settings: AttributesFormatString=|
      | twiddle_general_4mult
      | coeffs in BRAM
      | Bit growth:0
  butterfly4_0 : Subsystem
      settings: AttributesFormatString=|
      | twiddle_general_4mult
      | coeffs in BRAM
      | Bit growth:0
  butterfly4_1 : Subsystem
      settings: AttributesFormatString=|
      | twiddle_general_4mult
      | coeffs in BRAM
      | Bit growth:0
  butterfly4_2 : Subsystem
      settings: AttributesFormatString=|
      | twiddle_general_4mult
      | coeffs in BRAM
      | Bit growth:0
  butterfly4_3 : Subsystem
      settings: AttributesFormatString=|
      | twiddle_general_4mult
      | coeffs in BRAM
      | Bit growth:0
  butterfly4_4 : Subsystem
      settings: AttributesFormatString=|
      | twiddle_general_4mult
      | coeffs in BRAM
      | Bit growth:0
  butterfly4_5 : Subsystem
      settings: AttributesFormatString=|
      | twiddle_general_4mult
      | coeffs in BRAM
      | Bit growth:0
  butterfly4_6 : Subsystem
      settings: AttributesFormatString=|
      | twiddle_general_4mult
      | coeffs in BRAM
      | Bit growth:0
  butterfly4_7 : Subsystem
      settings: AttributesFormatString=|
      | twiddle_general_4mult
      | coeffs in BRAM
      | Bit growth:0
  node0_{0..15} (16 alike) : xbsIndex_r4/Delay  rst=off  infoeditControl=Selection of Reset will increase slice count due to use of real FFs and instead of SRLs  en=off  latency=0  dbl_ovrd=off  reg_retiming=off  block_type=delay
  node1_{0..15} (16 alike) : xbsIndex_r4/Delay  rst=off  infoeditControl=Selection of Reset will increase slice count due to use of real FFs and instead of SRLs  en=off  latency=0  dbl_ovrd=off  reg_retiming=off  block_type=delay
  node2_{0..15} (16 alike) : xbsIndex_r4/Delay  rst=off  infoeditControl=Selection of Reset will increase slice count due to use of real FFs and instead of SRLs  en=off  latency=0  dbl_ovrd=off  reg_retiming=off  block_type=delay
  node3_{0..15} (16 alike) : xbsIndex_r4/Delay  rst=off  infoeditControl=Selection of Reset will increase slice count due to use of real FFs and instead of SRLs  en=off  latency=0  dbl_ovrd=off  reg_retiming=off  block_type=delay
  node4_{0..15} (16 alike) : xbsIndex_r4/Delay  rst=off  infoeditControl=Selection of Reset will increase slice count due to use of real FFs and instead of SRLs  en=off  latency=0  dbl_ovrd=off  reg_retiming=off  block_type=delay
  of_{1..4} (4 alike) : xbsIndex_r4/Logical  logical_function=OR  inputs=8  en=off  latency=1  precision=Full  arith_type=Unsigned  n_bits=16  bin_pt=0  align_bp=on  dbl_ovrd=off  block_type=logical
  of_or : xbsIndex_r4/Logical  logical_function=OR  inputs=4  en=off  latency=0  precision=Full  arith_type=Unsigned  n_bits=16  bin_pt=0  align_bp=on  dbl_ovrd=off  block_type=logical
  slice0 : xbsIndex_r4/Slice  nbits=1  boolean_output=on  mode=Lower Bit Location + Width  bit1=0  base1=MSB of Input  bit0=0  base0=LSB of Input  dbl_ovrd=off  block_type=slice
  slice1 : xbsIndex_r4/Slice  nbits=1  boolean_output=on  mode=Lower Bit Location + Width  bit1=0  base1=MSB of Input  bit0=1  base0=LSB of Input  dbl_ovrd=off  block_type=slice
  slice2 : xbsIndex_r4/Slice  nbits=1  boolean_output=on  mode=Lower Bit Location + Width  bit1=0  base1=MSB of Input  bit0=2  base0=LSB of Input  dbl_ovrd=off  block_type=slice
  slice3 : xbsIndex_r4/Slice  nbits=1  boolean_output=on  mode=Lower Bit Location + Width  bit1=0  base1=MSB of Input  bit0=3  base0=LSB of Input  dbl_ovrd=off  block_type=slice
  sync_out : Outport
  dout_0 : Outport  Port=2
  dout_1 : Outport  Port=3
  dout_2 : Outport  Port=4
  dout_3 : Outport  Port=5
  dout_4 : Outport  Port=6
  dout_5 : Outport  Port=7
  dout_6 : Outport  Port=8
  dout_7 : Outport  Port=9
  dout_8 : Outport  Port=10
  dout_9 : Outport  Port=11
  dout_10 : Outport  Port=12
  dout_11 : Outport  Port=13
  dout_12 : Outport  Port=14
  dout_13 : Outport  Port=15
  dout_14 : Outport  Port=16
  dout_15 : Outport  Port=17
  of : Outport  Port=18
  wires:
  shift/1 -> slice3/1, slice2/1, slice1/1, slice0/1
  sync/1 -> butterfly1_7/3(sync), butterfly1_6/3(sync), butterfly1_5/3(sync), butterfly1_4/3(sync), butterfly1_3/3(sync), butterfly1_2/3(sync), butterfly1_1/3(sync), butterfly1_0/3(sync)
  slice0/1 -> butterfly1_7/4(shift), butterfly1_6/4(shift), butterfly1_5/4(shift), butterfly1_4/4(shift), butterfly1_3/4(shift), butterfly1_2/4(shift), butterfly1_1/4(shift), butterfly1_0/4(shift)
  slice1/1 -> butterfly2_7/4(shift), butterfly2_6/4(shift), butterfly2_5/4(shift), butterfly2_4/4(shift), butterfly2_3/4(shift), butterfly2_2/4(shift), butterfly2_1/4(shift), butterfly2_0/4(shift)
  slice2/1 -> butterfly3_7/4(shift), butterfly3_6/4(shift), butterfly3_5/4(shift), butterfly3_4/4(shift), butterfly3_3/4(shift), butterfly3_2/4(shift), butterfly3_1/4(shift), butterfly3_0/4(shift)
  slice3/1 -> butterfly4_7/4(shift), butterfly4_6/4(shift), butterfly4_5/4(shift), butterfly4_4/4(shift), butterfly4_3/4(shift), butterfly4_2/4(shift), butterfly4_1/4(shift), butterfly4_0/4(shift)
  butterfly4_0/4(sync_out) -> sync_out/1
  of_or/1 -> of/1
  of_4/1 -> of_or/4
  of_3/1 -> of_or/3
  of_2/1 -> of_or/2
  of_1/1 -> of_or/1
  butterfly4_7/3(of) -> of_4/8
  butterfly4_6/3(of) -> of_4/7
  butterfly4_5/3(of) -> of_4/6
  butterfly4_4/3(of) -> of_4/5
  butterfly4_3/3(of) -> of_4/4
  butterfly4_2/3(of) -> of_4/3
  butterfly4_1/3(of) -> of_4/2
  butterfly4_0/3(of) -> of_4/1
  butterfly4_7/2(a-bw) -> node4_15/1
  butterfly4_7/1(a+bw) -> node4_14/1
  butterfly3_7/4(sync_out) -> butterfly4_7/3(sync)
  node3_15/1 -> butterfly4_7/2(b)
  node3_14/1 -> butterfly4_7/1(a)
  butterfly4_6/2(a-bw) -> node4_13/1
  butterfly4_6/1(a+bw) -> node4_12/1
  butterfly3_6/4(sync_out) -> butterfly4_6/3(sync)
  node3_13/1 -> butterfly4_6/2(b)
  node3_12/1 -> butterfly4_6/1(a)
  butterfly4_5/2(a-bw) -> node4_11/1
  butterfly4_5/1(a+bw) -> node4_10/1
  butterfly3_5/4(sync_out) -> butterfly4_5/3(sync)
  node3_11/1 -> butterfly4_5/2(b)
  node3_10/1 -> butterfly4_5/1(a)
  butterfly4_4/2(a-bw) -> node4_9/1
  butterfly4_4/1(a+bw) -> node4_8/1
  butterfly3_4/4(sync_out) -> butterfly4_4/3(sync)
  node3_9/1 -> butterfly4_4/2(b)
  node3_8/1 -> butterfly4_4/1(a)
  butterfly4_3/2(a-bw) -> node4_7/1
  butterfly4_3/1(a+bw) -> node4_6/1
  butterfly3_3/4(sync_out) -> butterfly4_3/3(sync)
  node3_7/1 -> butterfly4_3/2(b)
  node3_6/1 -> butterfly4_3/1(a)
  butterfly4_2/2(a-bw) -> node4_5/1
  butterfly4_2/1(a+bw) -> node4_4/1
  butterfly3_2/4(sync_out) -> butterfly4_2/3(sync)
  node3_5/1 -> butterfly4_2/2(b)
  node3_4/1 -> butterfly4_2/1(a)
  butterfly4_1/2(a-bw) -> node4_3/1
  butterfly4_1/1(a+bw) -> node4_2/1
  butterfly3_1/4(sync_out) -> butterfly4_1/3(sync)
  node3_3/1 -> butterfly4_1/2(b)
  node3_2/1 -> butterfly4_1/1(a)
  butterfly4_0/2(a-bw) -> node4_1/1
  butterfly4_0/1(a+bw) -> node4_0/1
  butterfly3_0/4(sync_out) -> butterfly4_0/3(sync)
  node3_1/1 -> butterfly4_0/2(b)
  node3_0/1 -> butterfly4_0/1(a)
  butterfly3_7/3(of) -> of_3/8
  butterfly3_6/3(of) -> of_3/7
  butterfly3_5/3(of) -> of_3/6
  butterfly3_4/3(of) -> of_3/5
  butterfly3_3/3(of) -> of_3/4
  butterfly3_2/3(of) -> of_3/3
  butterfly3_1/3(of) -> of_3/2
  butterfly3_0/3(of) -> of_3/1
  butterfly3_7/2(a-bw) -> node3_15/1
  butterfly3_7/1(a+bw) -> node3_13/1
  butterfly2_7/4(sync_out) -> butterfly3_7/3(sync)
  node2_15/1 -> butterfly3_7/2(b)
  node2_13/1 -> butterfly3_7/1(a)
  butterfly3_6/2(a-bw) -> node3_14/1
  butterfly3_6/1(a+bw) -> node3_12/1
  butterfly2_6/4(sync_out) -> butterfly3_6/3(sync)
  node2_14/1 -> butterfly3_6/2(b)
  node2_12/1 -> butterfly3_6/1(a)
  butterfly3_5/2(a-bw) -> node3_11/1
  butterfly3_5/1(a+bw) -> node3_9/1
  butterfly2_5/4(sync_out) -> butterfly3_5/3(sync)
  node2_11/1 -> butterfly3_5/2(b)
  node2_9/1 -> butterfly3_5/1(a)
  butterfly3_4/2(a-bw) -> node3_10/1
  butterfly3_4/1(a+bw) -> node3_8/1
  butterfly2_4/4(sync_out) -> butterfly3_4/3(sync)
  node2_10/1 -> butterfly3_4/2(b)
  node2_8/1 -> butterfly3_4/1(a)
  butterfly3_3/2(a-bw) -> node3_7/1
  butterfly3_3/1(a+bw) -> node3_5/1
  butterfly2_3/4(sync_out) -> butterfly3_3/3(sync)
  node2_7/1 -> butterfly3_3/2(b)
  node2_5/1 -> butterfly3_3/1(a)
  butterfly3_2/2(a-bw) -> node3_6/1
  butterfly3_2/1(a+bw) -> node3_4/1
  butterfly2_2/4(sync_out) -> butterfly3_2/3(sync)
  node2_6/1 -> butterfly3_2/2(b)
  node2_4/1 -> butterfly3_2/1(a)
  butterfly3_1/2(a-bw) -> node3_3/1
  butterfly3_1/1(a+bw) -> node3_1/1
  butterfly2_1/4(sync_out) -> butterfly3_1/3(sync)
  node2_3/1 -> butterfly3_1/2(b)
  node2_1/1 -> butterfly3_1/1(a)
  butterfly3_0/2(a-bw) -> node3_2/1
  butterfly3_0/1(a+bw) -> node3_0/1
  butterfly2_0/4(sync_out) -> butterfly3_0/3(sync)
  node2_2/1 -> butterfly3_0/2(b)
  node2_0/1 -> butterfly3_0/1(a)
  butterfly2_7/3(of) -> of_2/8
  butterfly2_6/3(of) -> of_2/7
  butterfly2_5/3(of) -> of_2/6
  butterfly2_4/3(of) -> of_2/5
  butterfly2_3/3(of) -> of_2/4
  butterfly2_2/3(of) -> of_2/3
  butterfly2_1/3(of) -> of_2/2
  butterfly2_0/3(of) -> of_2/1
  butterfly2_7/2(a-bw) -> node2_15/1
  butterfly2_7/1(a+bw) -> node2_11/1
  butterfly1_7/4(sync_out) -> butterfly2_7/3(sync)
  node1_15/1 -> butterfly2_7/2(b)
  node1_11/1 -> butterfly2_7/1(a)
  butterfly2_6/2(a-bw) -> node2_14/1
  butterfly2_6/1(a+bw) -> node2_10/1
  butterfly1_6/4(sync_out) -> butterfly2_6/3(sync)
  node1_14/1 -> butterfly2_6/2(b)
  node1_10/1 -> butterfly2_6/1(a)
  butterfly2_5/2(a-bw) -> node2_13/1
  butterfly2_5/1(a+bw) -> node2_9/1
  butterfly1_5/4(sync_out) -> butterfly2_5/3(sync)
  node1_13/1 -> butterfly2_5/2(b)
  node1_9/1 -> butterfly2_5/1(a)
  butterfly2_4/2(a-bw) -> node2_12/1
  butterfly2_4/1(a+bw) -> node2_8/1
  butterfly1_4/4(sync_out) -> butterfly2_4/3(sync)
  node1_12/1 -> butterfly2_4/2(b)
  node1_8/1 -> butterfly2_4/1(a)
  butterfly2_3/2(a-bw) -> node2_7/1
  butterfly2_3/1(a+bw) -> node2_3/1
  butterfly1_3/4(sync_out) -> butterfly2_3/3(sync)
  node1_7/1 -> butterfly2_3/2(b)
  node1_3/1 -> butterfly2_3/1(a)
  butterfly2_2/2(a-bw) -> node2_6/1
  butterfly2_2/1(a+bw) -> node2_2/1
  butterfly1_2/4(sync_out) -> butterfly2_2/3(sync)
  node1_6/1 -> butterfly2_2/2(b)
  node1_2/1 -> butterfly2_2/1(a)
  butterfly2_1/2(a-bw) -> node2_5/1
  butterfly2_1/1(a+bw) -> node2_1/1
  butterfly1_1/4(sync_out) -> butterfly2_1/3(sync)
  node1_5/1 -> butterfly2_1/2(b)
  node1_1/1 -> butterfly2_1/1(a)
  butterfly2_0/2(a-bw) -> node2_4/1
  butterfly2_0/1(a+bw) -> node2_0/1
  butterfly1_0/4(sync_out) -> butterfly2_0/3(sync)
  node1_4/1 -> butterfly2_0/2(b)
  node1_0/1 -> butterfly2_0/1(a)
  butterfly1_7/3(of) -> of_1/8
  butterfly1_6/3(of) -> of_1/7
  butterfly1_5/3(of) -> of_1/6
  butterfly1_4/3(of) -> of_1/5
  butterfly1_3/3(of) -> of_1/4
  butterfly1_2/3(of) -> of_1/3
  butterfly1_1/3(of) -> of_1/2
  butterfly1_0/3(of) -> of_1/1
  butterfly1_7/2(a-bw) -> node1_15/1
  butterfly1_7/1(a+bw) -> node1_7/1
  node0_15/1 -> butterfly1_7/2(b)
  node0_7/1 -> butterfly1_7/1(a)
  butterfly1_6/2(a-bw) -> node1_14/1
  butterfly1_6/1(a+bw) -> node1_6/1
  node0_14/1 -> butterfly1_6/2(b)
  node0_6/1 -> butterfly1_6/1(a)
  butterfly1_5/2(a-bw) -> node1_13/1
  butterfly1_5/1(a+bw) -> node1_5/1
  node0_13/1 -> butterfly1_5/2(b)
  node0_5/1 -> butterfly1_5/1(a)
  butterfly1_4/2(a-bw) -> node1_12/1
  butterfly1_4/1(a+bw) -> node1_4/1
  node0_12/1 -> butterfly1_4/2(b)
  node0_4/1 -> butterfly1_4/1(a)
  butterfly1_3/2(a-bw) -> node1_11/1
  butterfly1_3/1(a+bw) -> node1_3/1
  node0_11/1 -> butterfly1_3/2(b)
  node0_3/1 -> butterfly1_3/1(a)
  butterfly1_2/2(a-bw) -> node1_10/1
  butterfly1_2/1(a+bw) -> node1_2/1
  node0_10/1 -> butterfly1_2/2(b)
  node0_2/1 -> butterfly1_2/1(a)
  butterfly1_1/2(a-bw) -> node1_9/1
  butterfly1_1/1(a+bw) -> node1_1/1
  node0_9/1 -> butterfly1_1/2(b)
  node0_1/1 -> butterfly1_1/1(a)
  butterfly1_0/2(a-bw) -> node1_8/1
  butterfly1_0/1(a+bw) -> node1_0/1
  node0_8/1 -> butterfly1_0/2(b)
  node0_0/1 -> butterfly1_0/1(a)
  node4_15/1 -> dout_15/1
  node4_14/1 -> dout_7/1
  node4_13/1 -> dout_11/1
  node4_12/1 -> dout_3/1
  node4_11/1 -> dout_13/1
  node4_10/1 -> dout_5/1
  node4_9/1 -> dout_9/1
  node4_8/1 -> dout_1/1
  node4_7/1 -> dout_14/1
  node4_6/1 -> dout_6/1
  node4_5/1 -> dout_10/1
  node4_4/1 -> dout_2/1
  node4_3/1 -> dout_12/1
  node4_2/1 -> dout_4/1
  node4_1/1 -> dout_8/1
  node4_0/1 -> dout_0/1
  din_15/1 -> node0_15/1
  din_14/1 -> node0_14/1
  din_13/1 -> node0_13/1
  din_12/1 -> node0_12/1
  din_11/1 -> node0_11/1
  din_10/1 -> node0_10/1
  din_9/1 -> node0_9/1
  din_8/1 -> node0_8/1
  din_7/1 -> node0_7/1
  din_6/1 -> node0_6/1
  din_5/1 -> node0_5/1
  din_4/1 -> node0_4/1
  din_3/1 -> node0_3/1
  din_2/1 -> node0_2/1
  din_1/1 -> node0_1/1
  din_0/1 -> node0_0/1

== system v01_aa_rii_16r4t11f_ver117sa/fft_wideband_real1/fft_unscrambler ==
  sync : Inport
  In1 : Inport  Port=2
  In2 : Inport  Port=3
  In3 : Inport  Port=4
  In4 : Inport  Port=5
  In5 : Inport  Port=6
  In6 : Inport  Port=7
  In7 : Inport  Port=8
  In8 : Inport  Port=9
  const : xbsIndex_r4/Constant  const=1  gui_display_data_type=Fixed-point  arith_type=Boolean  n_bits=1  bin_pt=0  preci_type=Single  exp_width=8  frac_width=24  explicit_period=on  period=1  equ=P=C  opselect=C  inp2=PCIN>>17  opr=+  inp1=P  carry=CIN  dbl_ovrd=off  block_type=constant
  reorder1 : Subsystem
      settings: AttributesFormatString=order=7
  square_transposer1 : Subsystem
      settings: AttributesFormatString=n_inputs=3
  sync_out : Outport
  Out1 : Outport  Port=2
  Out2 : Outport  Port=3
  Out3 : Outport  Port=4
  Out4 : Outport  Port=5
  Out5 : Outport  Port=6
  Out6 : Outport  Port=7
  Out7 : Outport  Port=8
  Out8 : Outport  Port=9
  wires:
  square_transposer1/9(Out8) -> reorder1/10(din7)  "xlsub2_reorder_In8"
  square_transposer1/8(Out7) -> reorder1/9(din6)  "xlsub2_reorder_In7"
  square_transposer1/7(Out6) -> reorder1/8(din5)  "xlsub2_reorder_In6"
  square_transposer1/6(Out5) -> reorder1/7(din4)  "xlsub2_reorder_In5"
  square_transposer1/5(Out4) -> reorder1/6(din3)  "xlsub2_reorder_In4"
  square_transposer1/4(Out3) -> reorder1/5(din2)  "xlsub2_reorder_In3"
  square_transposer1/3(Out2) -> reorder1/4(din1)  "xlsub2_reorder_In2"
  square_transposer1/2(Out1) -> reorder1/3(din0)  "xlsub2_reorder_In1"
  square_transposer1/1(sync_out) -> reorder1/1(sync)
  In8/1 -> square_transposer1/9(In8)
  In7/1 -> square_transposer1/8(In7)
  In6/1 -> square_transposer1/7(In6)
  In5/1 -> square_transposer1/6(In5)
  In4/1 -> square_transposer1/5(In4)
  In3/1 -> square_transposer1/4(In3)
  In2/1 -> square_transposer1/3(In2)
  In1/1 -> square_transposer1/2(In1)
  sync/1 -> square_transposer1/1(sync)
  reorder1/10(dout7) -> Out8/1
  reorder1/9(dout6) -> Out7/1
  reorder1/8(dout5) -> Out6/1
  reorder1/7(dout4) -> Out5/1
  reorder1/6(dout3) -> Out4/1
  reorder1/5(dout2) -> Out3/1
  reorder1/4(dout1) -> Out2/1
  reorder1/3(dout0) -> Out1/1
  reorder1/1(sync_out) -> sync_out/1
  const/1 -> reorder1/2(en)

== system v01_aa_rii_16r4t11f_ver117sa/fft_wideband_real1/in_del_4x0pol1 ==
  d : Inport
  q : Outport
  wires:
  d/1 -> q/1

== system v01_aa_rii_16r4t11f_ver117sa/fft_wideband_real1/in_del_4x0pol2 ==
  d : Inport
  q : Outport
  wires:
  d/1 -> q/1

== system v01_aa_rii_16r4t11f_ver117sa/fft_wideband_real1/in_del_4x0pol3 ==
  d : Inport
  q : Outport
  wires:
  d/1 -> q/1

== system v01_aa_rii_16r4t11f_ver117sa/fft_wideband_real1/in_del_4x0pol4 ==
  d : Inport
  q : Outport
  wires:
  d/1 -> q/1

== system v01_aa_rii_16r4t11f_ver117sa/fft_wideband_real1/in_del_4x1pol1 ==
  d : Inport
  q : Outport
  wires:
  d/1 -> q/1

== system v01_aa_rii_16r4t11f_ver117sa/fft_wideband_real1/in_del_4x1pol2 ==
  d : Inport
  q : Outport
  wires:
  d/1 -> q/1

== system v01_aa_rii_16r4t11f_ver117sa/fft_wideband_real1/in_del_4x1pol3 ==
  d : Inport
  q : Outport
  wires:
  d/1 -> q/1

== system v01_aa_rii_16r4t11f_ver117sa/fft_wideband_real1/in_del_4x1pol4 ==
  d : Inport
  q : Outport
  wires:
  d/1 -> q/1

== system v01_aa_rii_16r4t11f_ver117sa/fft_wideband_real1/in_del_4x2pol1 ==
  d : Inport
  q : Outport
  wires:
  d/1 -> q/1

== system v01_aa_rii_16r4t11f_ver117sa/fft_wideband_real1/in_del_4x2pol2 ==
  d : Inport
  q : Outport
  wires:
  d/1 -> q/1

== system v01_aa_rii_16r4t11f_ver117sa/fft_wideband_real1/in_del_4x2pol3 ==
  d : Inport
  q : Outport
  wires:
  d/1 -> q/1

== system v01_aa_rii_16r4t11f_ver117sa/fft_wideband_real1/in_del_4x2pol4 ==
  d : Inport
  q : Outport
  wires:
  d/1 -> q/1

== system v01_aa_rii_16r4t11f_ver117sa/fft_wideband_real1/in_del_4x3pol1 ==
  d : Inport
  q : Outport
  wires:
  d/1 -> q/1

== system v01_aa_rii_16r4t11f_ver117sa/fft_wideband_real1/in_del_4x3pol2 ==
  d : Inport
  q : Outport
  wires:
  d/1 -> q/1

== system v01_aa_rii_16r4t11f_ver117sa/fft_wideband_real1/in_del_4x3pol3 ==
  d : Inport
  q : Outport
  wires:
  d/1 -> q/1

== system v01_aa_rii_16r4t11f_ver117sa/fft_wideband_real1/in_del_4x3pol4 ==
  d : Inport
  q : Outport
  wires:
  d/1 -> q/1

== system v01_aa_rii_16r4t11f_ver117sa/fft_wideband_real1/in_del_sync_4x0 ==
  d : Inport
  q : Outport
  wires:
  d/1 -> q/1

== system v01_aa_rii_16r4t11f_ver117sa/fft_wideband_real1/in_del_sync_4x1 ==
  d : Inport
  q : Outport
  wires:
  d/1 -> q/1

== system v01_aa_rii_16r4t11f_ver117sa/fft_wideband_real1/in_del_sync_4x2 ==
  d : Inport
  q : Outport
  wires:
  d/1 -> q/1
